simulink model "ofdm_txrx_supermimo_coded"  (Simulink 7.7)
settings: Solver=VariableStepDiscrete  SolverMode=SingleTasking  StartTime=0.0  StopTime=2*simOnly_simLength  RelTol=1e-3
PostLoadFcn:
  | ofdm_rx_supermimo_init
InitFcn:
  | ofdm_rx_supermimo_init
StartFcn:
  | ofdm_rx_supermimo_init
values from neighbouring files (symbols this model uses):
  ActionID_AFTransmit = 31    [ofdm_tx_supermimo_init.m]
  ActionID_Disabled = 0    [ofdm_tx_supermimo_init.m]
  ActionID_SetFlagA = 62    [ofdm_tx_supermimo_init.m]
  ActionID_SetFlagB = 61    [ofdm_tx_supermimo_init.m]
  BER_RAM_init_size = 2048    [ofdm_tx_supermimo_init.m]
  channel_h = [1 0; 1 0] * 0.3    [ofdm_tx_supermimo_init.m]
  CPLength = 16    [ofdm_tx_supermimo_init.m]
  longCorr_coef_i = [-4*real(LTS) 0]    [ofdm_rx_supermimo_init.m]
  longCorr_coef_q = [4*imag(LTS) 0]    [ofdm_rx_supermimo_init.m]
  max_CP_length = 16    [ofdm_tx_supermimo_init.m]
  max_num_baseRateSymbols = 31    [ofdm_tx_supermimo_init.m]
  max_num_subcarriers = 64    [ofdm_tx_supermimo_init.m]
  max_num_trainingSymbols = 15    [ofdm_tx_supermimo_init.m]
  max_numBytes = 16384    [ofdm_tx_supermimo_init.m]
  max_OFDM_symbols = 1023    [ofdm_tx_supermimo_init.m]
  modConstellation_qam16 = .75*[1 3 -1 -3]./3    [ofdm_tx_supermimo_init.m]
  modConstellation_qam64 = 0.875*[3 1 5 7 -3 -1 -5 -7]./7    [ofdm_tx_supermimo_init.m]
  modConstellation_qpsk = [1 -1]./sqrt(2)    [ofdm_tx_supermimo_init.m]
  numBaseRateSymbols = numBaseRateSymbols *2    [ofdm_tx_supermimo_init.m]
  numSubcarriers = 64    [ofdm_tx_supermimo_init.m]
  numTrainingSymbols = 2    [ofdm_tx_supermimo_init.m]
  pktTiming_count_payloadStart = pktTiming_count_lsStart + pktTiming_ls_num*pktTiming_ls_length    [ofdm_rx_supermimo_init.m]
  preamble = preamble_scale*[shortsyms_10 longsyms_2]    [ofdm_tx_supermimo_init.m]
  preamble_ROM = preamble_I_ROM + preamble_Q_ROM*2^16    [ofdm_tx_supermimo_init.m]
  RAM_init_size = max_numBytes    (= 16384)    [ofdm_tx_supermimo_init.m]
  RxDataScrambling_Seq = [40 198 78 63 82 173 102 245 48 111 172 115 147 230 216 93 72 65 62 2 205 242 122 90 128 83 105 97 73 10 5 252]    [ofdm_tx_supermimo_init.m]
  shortSymbol_time = shortSymbol_time(1:16).'    [ofdm_tx_supermimo_init.m]
  simOnly_numSamples = length(preamble)+( (numSubcarriers+CPLength)*(numTrainingSymbols + numBaseRateSymbols + numFullRateSymbols/2) )    [ofdm_tx_supermimo_init.m]
  train = [train train]    [ofdm_tx_supermimo_init.m]
  TxDataScrambling_Seq = [40 198 78 63 82 173 102 245 48 111 172 115 147 230 216 93 72 65 62 2 205 242 122 90 128 83 105 97 73 10 5 252]    [ofdm_tx_supermimo_init.m]
undefined symbols (not defined in the file or in its folder): AntA, AntB, BaseRate, Down, gcbh, numBuffs, numBytes, Up, xlBlockLoadCallback, xlGetNormalizedPeriod, xlipgetpartsetting
libraries: xbsIndex_r4 x5430
(part 9 of 49)

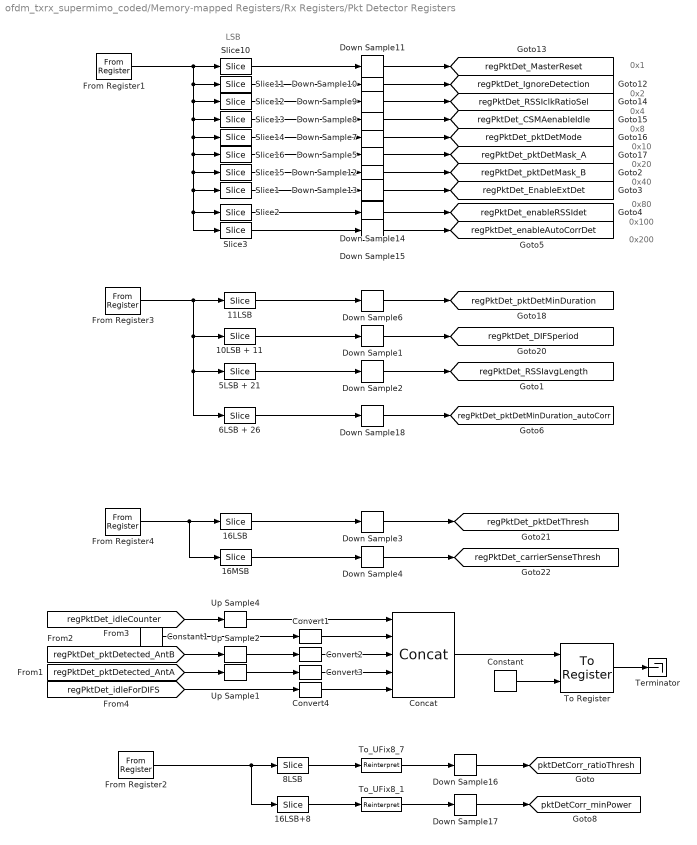
== system ofdm_txrx_supermimo_coded/Memory-mapped Registers/Rx Registers/Pkt Detector Registers ==
  note: 0x1
  note: 0x2
  note: 0x4
  note: 0x8
  note: 0x10
  note: LSB
  note: 0x20
  note: 0x40
  note: 0x80
  note: 0x100
  note: 0x200
  10LSB + 11 : xbsIndex_r4/Slice  nbits=10  boolean_output=off  mode=Lower Bit Location + Width  bit1=0  base1=MSB of Input  bit0=11  base0=LSB of Input  dbl_ovrd=off  block_type=slice
      settings: infoedit="Extracts a given range of bits from each input sample and presents it at the output.  The output type is ordinarily unsigned with binary point at zero, but can be Boolean when the slice is one bit wide.<P><P>Hardware notes: In hardware this block costs nothing."  block_version=VER_STRING_GOES_HERE
  11LSB : xbsIndex_r4/Slice  nbits=11  boolean_output=off  mode=Lower Bit Location + Width  bit1=0  base1=MSB of Input  bit0=0  base0=LSB of Input  dbl_ovrd=off  block_type=slice
      settings: infoedit="Extracts a given range of bits from each input sample and presents it at the output.  The output type is ordinarily unsigned with binary point at zero, but can be Boolean when the slice is one bit wide.<P><P>Hardware notes: In hardware this block costs nothing."  block_version=VER_STRING_GOES_HERE
  16LSB : xbsIndex_r4/Slice  nbits=16  boolean_output=off  mode=Lower Bit Location + Width  bit1=0  base1=MSB of Input  bit0=0  base0=LSB of Input  dbl_ovrd=off  block_type=slice
      settings: infoedit="Extracts a given range of bits from each input sample and presents it at the output.  The output type is ordinarily unsigned with binary point at zero, but can be Boolean when the slice is one bit wide.<P><P>Hardware notes: In hardware this block costs nothing."  block_version=VER_STRING_GOES_HERE
  16LSB+8 : xbsIndex_r4/Slice  nbits=16  boolean_output=off  mode=Lower Bit Location + Width  bit1=0  base1=MSB of Input  bit0=8  base0=LSB of Input  dbl_ovrd=off  block_type=slice
      settings: infoedit="Extracts a given range of bits from each input sample and presents it at the output.  The output type is ordinarily unsigned with binary point at zero, but can be Boolean when the slice is one bit wide.<P><P>Hardware notes: In hardware this block costs nothing."  block_version=VER_STRING_GOES_HERE
  16MSB : xbsIndex_r4/Slice  nbits=16  boolean_output=off  mode=Lower Bit Location + Width  bit1=0  base1=MSB of Input  bit0=16  base0=LSB of Input  dbl_ovrd=off  block_type=slice
      settings: infoedit="Extracts a given range of bits from each input sample and presents it at the output.  The output type is ordinarily unsigned with binary point at zero, but can be Boolean when the slice is one bit wide.<P><P>Hardware notes: In hardware this block costs nothing."  block_version=VER_STRING_GOES_HERE
  5LSB + 21 : xbsIndex_r4/Slice  nbits=5  boolean_output=off  mode=Lower Bit Location + Width  bit1=0  base1=MSB of Input  bit0=21  base0=LSB of Input  dbl_ovrd=off  block_type=slice
      settings: infoedit="Extracts a given range of bits from each input sample and presents it at the output.  The output type is ordinarily unsigned with binary point at zero, but can be Boolean when the slice is one bit wide.<P><P>Hardware notes: In hardware this block costs nothing."  block_version=VER_STRING_GOES_HERE
  6LSB + 26 : xbsIndex_r4/Slice  nbits=6  boolean_output=off  mode=Lower Bit Location + Width  bit1=0  base1=MSB of Input  bit0=26  base0=LSB of Input  dbl_ovrd=off  block_type=slice
      settings: block_version=VER_STRING_GOES_HERE
  8LSB : xbsIndex_r4/Slice  nbits=8  boolean_output=off  mode=Lower Bit Location + Width  bit1=0  base1=MSB of Input  bit0=0  base0=LSB of Input  dbl_ovrd=off  block_type=slice
      settings: infoedit="Extracts a given range of bits from each input sample and presents it at the output.  The output type is ordinarily unsigned with binary point at zero, but can be Boolean when the slice is one bit wide.<P><P>Hardware notes: In hardware this block costs nothing."  block_version=VER_STRING_GOES_HERE
  Concat : xbsIndex_r4/Concat  num_inputs=5  dbl_ovrd=off  block_type=concat
      settings: block_version=10.1.3
  Constant : xbsIndex_r4/Constant  const=1  gui_display_data_type=Fixed-point  arith_type=Boolean  n_bits=16  bin_pt=14  preci_type=Single  exp_width=8  frac_width=24  explicit_period=off  period=1  equ=P=C  opselect=C  inp2=PCIN>>17  opr=+  inp1=P  carry=CIN  dbl_ovrd=off  block_type=constant
  Constant1 : xbsIndex_r4/Constant  const=0  gui_display_data_type=Fixed-point  arith_type=Unsigned  n_bits=1  bin_pt=0  preci_type=Single  exp_width=8  frac_width=24  explicit_period=off  period=1  equ=P=C  opselect=C  inp2=PCIN>>17  opr=+  inp1=P  carry=CIN  dbl_ovrd=off  block_type=constant
  Convert{1..4} (4 alike) : xbsIndex_r4/Convert  gui_display_data_type=Fixed-point  arith_type=Unsigned  n_bits=1  bin_pt=0  float_type=Single  exp_bits=8  fraction_bits=24  quantization=Truncate  overflow=Wrap  en=off  latency=0  dbl_ovrd=off  pipeline=off  block_type=convert
      settings: block_version=10.1.3
  Down Sample{1..18} (18 alike) : xbsIndex_r4/Down Sample  sample_ratio=4  sample_phase="Last Value of Frame  (most efficient)"  en=off  latency=1  dbl_ovrd=off  block_type=dsamp
      settings: block_version=VER_STRING_GOES_HERE
  From Register1 : xbsIndex_r4/From Register  shared_memory_name='pktDet_controlBits'  init=pktDet_controlBits  period=1  ownership=Locally owned and initialized  gui_display_data_type=Fixed-point  arith_type=Unsigned  n_bits=32  bin_pt=0  preci_type=Single  dbl_ovrd=off  block_type=fromreg
      settings: AttributesFormatString=<< %<shared_memory_name> >>  infoedit="Register block that reads data to a shared memory register.  Delay of one sample period."
  From Register2 : xbsIndex_r4/From Register  shared_memory_name='pktDet_autoCorrParams'  init=rxReg_pktDetCorr  period=1  ownership=Locally owned and initialized  gui_display_data_type=Fixed-point  arith_type=Unsigned  n_bits=32  bin_pt=0  preci_type=Single  dbl_ovrd=off  block_type=fromreg
      settings: AttributesFormatString=<< %<shared_memory_name> >>  infoedit="Register block that reads data to a shared memory register.  Delay of one sample period."
  From Register3 : xbsIndex_r4/From Register  shared_memory_name='pktDet_durations'  init=(48 * 2^0) + (1000 * 2^11) + (16 * 2^21) + (1 * 2^26)  period=1  ownership=Locally owned and initialized  gui_display_data_type=Fixed-point  arith_type=Unsigned  n_bits=32  bin_pt=0  preci_type=Single  dbl_ovrd=off  block_type=fromreg
      settings: AttributesFormatString=<< %<shared_memory_name> >>  infoedit="Register block that reads data to a shared memory register.  Delay of one sample period."
  From Register4 : xbsIndex_r4/From Register  shared_memory_name='pktDet_thresholds'  init=(3000 * 2^0) + (3000 * 2^16)  period=1  ownership=Locally owned and initialized  gui_display_data_type=Fixed-point  arith_type=Unsigned  n_bits=32  bin_pt=0  preci_type=Single  dbl_ovrd=off  block_type=fromreg
      settings: AttributesFormatString=<< %<shared_memory_name> >>  infoedit="Register block that reads data to a shared memory register.  Delay of one sample period."
  Slice1 : xbsIndex_r4/Slice  nbits=1  boolean_output=on  mode=Lower Bit Location + Width  bit1=0  base1=MSB of Input  bit0=7  base0=LSB of Input  dbl_ovrd=off  block_type=slice
      settings: infoedit="Extracts a given range of bits from each input sample and presents it at the output.  The output type is ordinarily unsigned with binary point at zero, but can be Boolean when the slice is one bit wide.<P><P>Hardware notes: In hardware this block costs nothing."  block_version=VER_STRING_GOES_HERE
  Slice10 : xbsIndex_r4/Slice  nbits=1  boolean_output=on  mode=Lower Bit Location + Width  bit1=0  base1=MSB of Input  bit0=0  base0=LSB of Input  dbl_ovrd=off  block_type=slice
      settings: infoedit="Extracts a given range of bits from each input sample and presents it at the output.  The output type is ordinarily unsigned with binary point at zero, but can be Boolean when the slice is one bit wide.<P><P>Hardware notes: In hardware this block costs nothing."  block_version=VER_STRING_GOES_HERE
  Slice11 : xbsIndex_r4/Slice  nbits=1  boolean_output=on  mode=Lower Bit Location + Width  bit1=0  base1=MSB of Input  bit0=1  base0=LSB of Input  dbl_ovrd=off  block_type=slice
      settings: infoedit="Extracts a given range of bits from each input sample and presents it at the output.  The output type is ordinarily unsigned with binary point at zero, but can be Boolean when the slice is one bit wide.<P><P>Hardware notes: In hardware this block costs nothing."  block_version=VER_STRING_GOES_HERE
  Slice12 : xbsIndex_r4/Slice  nbits=1  boolean_output=on  mode=Lower Bit Location + Width  bit1=0  base1=MSB of Input  bit0=2  base0=LSB of Input  dbl_ovrd=off  block_type=slice
      settings: infoedit="Extracts a given range of bits from each input sample and presents it at the output.  The output type is ordinarily unsigned with binary point at zero, but can be Boolean when the slice is one bit wide.<P><P>Hardware notes: In hardware this block costs nothing."  block_version=VER_STRING_GOES_HERE
  Slice13 : xbsIndex_r4/Slice  nbits=1  boolean_output=on  mode=Lower Bit Location + Width  bit1=0  base1=MSB of Input  bit0=3  base0=LSB of Input  dbl_ovrd=off  block_type=slice
      settings: infoedit="Extracts a given range of bits from each input sample and presents it at the output.  The output type is ordinarily unsigned with binary point at zero, but can be Boolean when the slice is one bit wide.<P><P>Hardware notes: In hardware this block costs nothing."  block_version=VER_STRING_GOES_HERE
  Slice14 : xbsIndex_r4/Slice  nbits=1  boolean_output=on  mode=Lower Bit Location + Width  bit1=0  base1=MSB of Input  bit0=4  base0=LSB of Input  dbl_ovrd=off  block_type=slice
      settings: infoedit="Extracts a given range of bits from each input sample and presents it at the output.  The output type is ordinarily unsigned with binary point at zero, but can be Boolean when the slice is one bit wide.<P><P>Hardware notes: In hardware this block costs nothing."  block_version=VER_STRING_GOES_HERE
  Slice15 : xbsIndex_r4/Slice  nbits=1  boolean_output=on  mode=Lower Bit Location + Width  bit1=0  base1=MSB of Input  bit0=6  base0=LSB of Input  dbl_ovrd=off  block_type=slice
      settings: infoedit="Extracts a given range of bits from each input sample and presents it at the output.  The output type is ordinarily unsigned with binary point at zero, but can be Boolean when the slice is one bit wide.<P><P>Hardware notes: In hardware this block costs nothing."  block_version=VER_STRING_GOES_HERE
  Slice16 : xbsIndex_r4/Slice  nbits=1  boolean_output=on  mode=Lower Bit Location + Width  bit1=0  base1=MSB of Input  bit0=5  base0=LSB of Input  dbl_ovrd=off  block_type=slice
      settings: infoedit="Extracts a given range of bits from each input sample and presents it at the output.  The output type is ordinarily unsigned with binary point at zero, but can be Boolean when the slice is one bit wide.<P><P>Hardware notes: In hardware this block costs nothing."  block_version=VER_STRING_GOES_HERE
  Slice2 : xbsIndex_r4/Slice  nbits=1  boolean_output=on  mode=Lower Bit Location + Width  bit1=0  base1=MSB of Input  bit0=8  base0=LSB of Input  dbl_ovrd=off  block_type=slice
      settings: infoedit="Extracts a given range of bits from each input sample and presents it at the output.  The output type is ordinarily unsigned with binary point at zero, but can be Boolean when the slice is one bit wide.<P><P>Hardware notes: In hardware this block costs nothing."  block_version=VER_STRING_GOES_HERE
  Slice3 : xbsIndex_r4/Slice  nbits=1  boolean_output=on  mode=Lower Bit Location + Width  bit1=0  base1=MSB of Input  bit0=9  base0=LSB of Input  dbl_ovrd=off  block_type=slice
      settings: infoedit="Extracts a given range of bits from each input sample and presents it at the output.  The output type is ordinarily unsigned with binary point at zero, but can be Boolean when the slice is one bit wide.<P><P>Hardware notes: In hardware this block costs nothing."  block_version=VER_STRING_GOES_HERE
  Terminator : Terminator
  To Register : xbsIndex_r4/To Register  shared_memory_name='pktDet_status'  init=0  ownership=Locally owned and initialized  explicit_data_type=on  gui_display_data_type=Fixed-point  arith_type=Unsigned  n_bits=14  bin_pt=0  preci_type=Single  dbl_ovrd=off  block_type=toreg
      settings: AttributesFormatString=<< %<shared_memory_name> >>  infoedit="Register block that writes data to a shared memory register.  Delay of one sample period."  block_version=10.1.3
  To_UFix8_1 : xbsIndex_r4/Reinterpret  force_arith_type=on  arith_type=Unsigned  force_bin_pt=on  bin_pt=8  block_type=cast
      settings: infoedit="Changes type of samples without altering their binary representation.<P><P>Hardware notes: In hardware this block costs nothing.<P><P>Example:  Suppose the input is 6 bits wide, signed, with 2 fractional bits, and the output is forced to unsigned with 0 fractional bits.  Then an input of -2.0 (1110.00 in binary 2's complement) becomes an output of 56 (111000 in binary)."  block_version=VER_STRING_GOES_HERE
  To_UFix8_7 : xbsIndex_r4/Reinterpret  force_arith_type=on  arith_type=Unsigned  force_bin_pt=on  bin_pt=7  block_type=cast
      settings: infoedit="Changes type of samples without altering their binary representation.<P><P>Hardware notes: In hardware this block costs nothing.<P><P>Example:  Suppose the input is 6 bits wide, signed, with 2 fractional bits, and the output is forced to unsigned with 0 fractional bits.  Then an input of -2.0 (1110.00 in binary 2's complement) becomes an output of 56 (111000 in binary)."  block_version=VER_STRING_GOES_HERE
  Up Sample1 : xbsIndex_r4/Up Sample  sample_ratio=4  copy_samples=on  en=off  latency=0  dbl_ovrd=off  block_type=usamp
      settings: infoedit="Up samples input data.  Inserted values  can be zeros or copies of the most recent input sample.<P><P>Hardware notes: No hardware is needed if inserted values are copies of the input sample; otherwise, a mux and single bit flip-flop are used."  block_version=8.2
  Up Sample2 : xbsIndex_r4/Up Sample  sample_ratio=4  copy_samples=on  en=off  latency=0  dbl_ovrd=off  block_type=usamp
      settings: infoedit="Up samples input data.  Inserted values  can be zeros or copies of the most recent input sample.<P><P>Hardware notes: No hardware is needed if inserted values are copies of the input sample; otherwise, a mux and single bit flip-flop are used."  block_version=8.2
  Up Sample4 : xbsIndex_r4/Up Sample  sample_ratio=4  copy_samples=on  en=off  latency=0  dbl_ovrd=off  block_type=usamp
      settings: infoedit="Up samples input data.  Inserted values  can be zeros or copies of the most recent input sample.<P><P>Hardware notes: No hardware is needed if inserted values are copies of the input sample; otherwise, a mux and single bit flip-flop are used."  block_version=8.2
  tag scope: pktDetCorr_minPower=global, pktDetCorr_ratioThresh=global, regPktDet_CSMAenableIdle=global, regPktDet_DIFSperiod=global, regPktDet_EnableExtDet=global, regPktDet_IgnoreDetection=global, regPktDet_MasterReset=global, regPktDet_RSSIavgLength=global, regPktDet_RSSIclkRatioSel=global, regPktDet_carrierSenseThresh=global, regPktDet_enableAutoCorrDet=global, regPktDet_enableRSSIdet=global, regPktDet_pktDetMask_A=global, regPktDet_pktDetMask_B=global, regPktDet_pktDetMinDuration=global, regPktDet_pktDetMinDuration_autoCorr=global, regPktDet_pktDetMode=global, regPktDet_pktDetThresh=global
  wires:
  From Register1/1 -> Slice10/1, Slice11/1, Slice12/1, Slice13/1, Slice14/1, Slice16/1, Slice15/1, Slice1/1, Slice2/1, Slice3/1
  Slice10/1 -> Down Sample11/1
  Slice11/1 -> Down Sample10/1
  Slice12/1 -> Down Sample9/1
  Slice13/1 -> Down Sample8/1
  Slice14/1 -> Down Sample7/1
  From Register3/1 -> 11LSB/1, 10LSB + 11/1, 5LSB + 21/1, 6LSB + 26/1
  11LSB/1 -> Down Sample6/1
  10LSB + 11/1 -> Down Sample1/1
  Down Sample6/1 -> [regPktDet_pktDetMinDuration]
  Down Sample1/1 -> [regPktDet_DIFSperiod]
  5LSB + 21/1 -> Down Sample2/1
  Down Sample2/1 -> [regPktDet_RSSIavgLength]
  From Register4/1 -> 16LSB/1, 16MSB/1
  16LSB/1 -> Down Sample3/1
  Down Sample3/1 -> [regPktDet_pktDetThresh]
  16MSB/1 -> Down Sample4/1
  Down Sample4/1 -> [regPktDet_carrierSenseThresh]
  Down Sample5/1 -> [regPktDet_pktDetMask_A]
  Down Sample7/1 -> [regPktDet_pktDetMode]
  Down Sample8/1 -> [regPktDet_CSMAenableIdle]
  Down Sample9/1 -> [regPktDet_RSSIclkRatioSel]
  Down Sample10/1 -> [regPktDet_IgnoreDetection]
  Down Sample11/1 -> [regPktDet_MasterReset]
  Slice16/1 -> Down Sample5/1
  Slice15/1 -> Down Sample12/1
  Down Sample12/1 -> [regPktDet_pktDetMask_B]
  Constant/1 -> To Register/2
  Concat/1 -> To Register/1
  [regPktDet_idleForDIFS] -> Convert4/1
  [regPktDet_pktDetected_AntA] -> Up Sample1/1
  [regPktDet_pktDetected_AntB] -> Up Sample2/1
  Constant1/1 -> Convert1/1
  [regPktDet_idleCounter] -> Up Sample4/1
  Up Sample4/1 -> Concat/1
  Up Sample2/1 -> Convert2/1
  Up Sample1/1 -> Convert3/1
  Convert1/1 -> Concat/2
  Convert2/1 -> Concat/3
  Convert3/1 -> Concat/4
  Convert4/1 -> Concat/5
  To Register/1 -> Terminator/1
  Slice1/1 -> Down Sample13/1
  Down Sample13/1 -> [regPktDet_EnableExtDet]
  Slice2/1 -> Down Sample14/1
  Down Sample14/1 -> [regPktDet_enableRSSIdet]
  Slice3/1 -> Down Sample15/1
  Down Sample15/1 -> [regPktDet_enableAutoCorrDet]
  From Register2/1 -> 16LSB+8/1, 8LSB/1
  8LSB/1 -> To_UFix8_7/1
  To_UFix8_7/1 -> Down Sample16/1
  16LSB+8/1 -> To_UFix8_1/1
  Down Sample16/1 -> [pktDetCorr_ratioThresh]
  Down Sample17/1 -> [pktDetCorr_minPower]
  To_UFix8_1/1 -> Down Sample17/1
  6LSB + 26/1 -> Down Sample18/1
  Down Sample18/1 -> [regPktDet_pktDetMinDuration_autoCorr]

== system ofdm_txrx_supermimo_coded/Memory-mapped Registers/Rx Registers/UFix32  ==
  I : Inport
  Convert : xbsIndex_r4/Convert  gui_display_data_type=Fixed-point  arith_type=Unsigned  n_bits=32  bin_pt=0  float_type=Single  exp_bits=8  fraction_bits=24  quantization=Truncate  overflow=Wrap  en=off  latency=0  dbl_ovrd=off  pipeline=off  block_type=convert
  Reinterpret : xbsIndex_r4/Reinterpret  force_arith_type=on  arith_type=Unsigned  force_bin_pt=on  bin_pt=0  block_type=reinterpret
  O : Outport
  wires:
  Reinterpret/1 -> Convert/1
  Convert/1 -> O/1
  I/1 -> Reinterpret/1

== system ofdm_txrx_supermimo_coded/Memory-mapped Registers/Rx Registers/UFix32 1 ==
  I : Inport
  Convert : xbsIndex_r4/Convert  gui_display_data_type=Fixed-point  arith_type=Unsigned  n_bits=32  bin_pt=0  float_type=Single  exp_bits=8  fraction_bits=24  quantization=Truncate  overflow=Wrap  en=off  latency=0  dbl_ovrd=off  pipeline=off  block_type=convert
  Reinterpret : xbsIndex_r4/Reinterpret  force_arith_type=on  arith_type=Unsigned  force_bin_pt=on  bin_pt=0  block_type=reinterpret
  O : Outport
  wires:
  I/1 -> Reinterpret/1
  Convert/1 -> O/1
  Reinterpret/1 -> Convert/1

== system ofdm_txrx_supermimo_coded/Memory-mapped Registers/Rx Registers/UFix32 2 ==
  I : Inport
  Convert : xbsIndex_r4/Convert  gui_display_data_type=Fixed-point  arith_type=Unsigned  n_bits=32  bin_pt=0  float_type=Single  exp_bits=8  fraction_bits=24  quantization=Truncate  overflow=Wrap  en=off  latency=0  dbl_ovrd=off  pipeline=off  block_type=convert
  Reinterpret : xbsIndex_r4/Reinterpret  force_arith_type=on  arith_type=Unsigned  force_bin_pt=on  bin_pt=0  block_type=reinterpret
  O : Outport
  wires:
  Reinterpret/1 -> Convert/1
  Convert/1 -> O/1
  I/1 -> Reinterpret/1

== system ofdm_txrx_supermimo_coded/Memory-mapped Registers/Rx Registers/UFix32 3 ==
  I : Inport
  Convert : xbsIndex_r4/Convert  gui_display_data_type=Fixed-point  arith_type=Unsigned  n_bits=32  bin_pt=0  float_type=Single  exp_bits=8  fraction_bits=24  quantization=Truncate  overflow=Wrap  en=off  latency=0  dbl_ovrd=off  pipeline=off  block_type=convert
  Reinterpret : xbsIndex_r4/Reinterpret  force_arith_type=on  arith_type=Unsigned  force_bin_pt=on  bin_pt=0  block_type=reinterpret
  O : Outport
  wires:
  I/1 -> Reinterpret/1
  Convert/1 -> O/1
  Reinterpret/1 -> Convert/1

== system ofdm_txrx_supermimo_coded/Memory-mapped Registers/Rx Registers/UFix32 4 ==
  I : Inport
  Convert : xbsIndex_r4/Convert  gui_display_data_type=Fixed-point  arith_type=Unsigned  n_bits=32  bin_pt=0  float_type=Single  exp_bits=8  fraction_bits=24  quantization=Truncate  overflow=Wrap  en=off  latency=0  dbl_ovrd=off  pipeline=off  block_type=convert
  Reinterpret : xbsIndex_r4/Reinterpret  force_arith_type=on  arith_type=Unsigned  force_bin_pt=on  bin_pt=0  block_type=reinterpret
  O : Outport
  wires:
  Reinterpret/1 -> Convert/1
  Convert/1 -> O/1
  I/1 -> Reinterpret/1

== system ofdm_txrx_supermimo_coded/Memory-mapped Registers/Rx Registers/UFix32 5 ==
  I : Inport
  Convert : xbsIndex_r4/Convert  gui_display_data_type=Fixed-point  arith_type=Unsigned  n_bits=32  bin_pt=0  float_type=Single  exp_bits=8  fraction_bits=24  quantization=Truncate  overflow=Wrap  en=off  latency=0  dbl_ovrd=off  pipeline=off  block_type=convert
  Reinterpret : xbsIndex_r4/Reinterpret  force_arith_type=on  arith_type=Unsigned  force_bin_pt=on  bin_pt=0  block_type=reinterpret
  O : Outport
  wires:
  I/1 -> Reinterpret/1
  Convert/1 -> O/1
  Reinterpret/1 -> Convert/1

== system ofdm_txrx_supermimo_coded/Memory-mapped Registers/Rx Registers/UFix32 6 ==
  I : Inport
  Convert : xbsIndex_r4/Convert  gui_display_data_type=Fixed-point  arith_type=Unsigned  n_bits=32  bin_pt=0  float_type=Single  exp_bits=8  fraction_bits=24  quantization=Truncate  overflow=Wrap  en=off  latency=0  dbl_ovrd=off  pipeline=off  block_type=convert
  Reinterpret : xbsIndex_r4/Reinterpret  force_arith_type=on  arith_type=Unsigned  force_bin_pt=on  bin_pt=0  block_type=reinterpret
  O : Outport
  wires:
  Reinterpret/1 -> Convert/1
  Convert/1 -> O/1
  I/1 -> Reinterpret/1

== system ofdm_txrx_supermimo_coded/Memory-mapped Registers/Tx Registers/Control Bits Slices ==
  note: LSB
  note: 0x1
  note: 0x2
  note: 0x4
  note: 0x8
  note: 0xF0
  note: 0x200
  note: 0x400
  note: 0x100
  note: 0x800
  note: 0x1000
  note: 0x2000
  note: 0x4000
  note: 0x8000
  note: 0x1_0000
  note: 0x2_0000
  note: 0x4_0000
  note: 0x8_0000
  note: 0x3F0_0000
  32b : Inport
  Down Sample1 : xbsIndex_r4/Down Sample  sample_ratio=2  sample_phase="Last Value of Frame  (most efficient)"  en=off  latency=1  dbl_ovrd=off  block_type=dsamp
      settings: block_version=VER_STRING_GOES_HERE
  Down Sample3 : xbsIndex_r4/Down Sample  sample_ratio=2  sample_phase="Last Value of Frame  (most efficient)"  en=off  latency=1  dbl_ovrd=off  block_type=dsamp
      settings: block_version=VER_STRING_GOES_HERE
  Reinterpret : xbsIndex_r4/Reinterpret  force_arith_type=on  arith_type="Signed  (2's comp)"  force_bin_pt=off  bin_pt=13  block_type=reinterpret
      settings: infoedit="Changes signal type without altering the binary representation.   You can changed the signal between signed and unsigned, and relocate the binary point.<P><P>Hardware notes: In hardware this block costs nothing.<P><P>Example:  Suppose the input is 6 bits wide, signed, with 2 fractional bits, and the output is forced to unsigned with 0 fractional bits.  Then an input of -2.0 (1110.00 in binary 2's complement) becomes an output of 56 (111000 in binary)."  block_version=10.1
  Slice1 : xbsIndex_r4/Slice  nbits=1  boolean_output=on  mode=Lower Bit Location + Width  bit1=0  base1=MSB of Input  bit0=0  base0=LSB of Input  dbl_ovrd=off  block_type=slice
      settings: infoedit="Extracts a given range of bits from each input sample and presents it at the output.  The output type is ordinarily unsigned with binary point at zero, but can be Boolean when the slice is one bit wide.<P><P>Hardware notes: In hardware this block costs nothing."  block_version=VER_STRING_GOES_HERE
  Slice10 : xbsIndex_r4/Slice  nbits=1  boolean_output=on  mode=Lower Bit Location + Width  bit1=0  base1=MSB of Input  bit0=15  base0=LSB of Input  dbl_ovrd=off  block_type=slice
      settings: infoedit="Extracts a given range of bits from each input sample and presents it at the output.  The output type is ordinarily unsigned with binary point at zero, but can be Boolean when the slice is one bit wide.<P><P>Hardware notes: In hardware this block costs nothing."  block_version=VER_STRING_GOES_HERE
  Slice11 : xbsIndex_r4/Slice  nbits=1  boolean_output=off  mode=Lower Bit Location + Width  bit1=0  base1=MSB of Input  bit0=12  base0=LSB of Input  dbl_ovrd=off  block_type=slice
      settings: infoedit="Extracts a given range of bits from each input sample and presents it at the output.  The output type is ordinarily unsigned with binary point at zero, but can be Boolean when the slice is one bit wide.<P><P>Hardware notes: In hardware this block costs nothing."  block_version=VER_STRING_GOES_HERE
  Slice12 : xbsIndex_r4/Slice  nbits=1  boolean_output=off  mode=Lower Bit Location + Width  bit1=0  base1=MSB of Input  bit0=13  base0=LSB of Input  dbl_ovrd=off  block_type=slice
      settings: infoedit="Extracts a given range of bits from each input sample and presents it at the output.  The output type is ordinarily unsigned with binary point at zero, but can be Boolean when the slice is one bit wide.<P><P>Hardware notes: In hardware this block costs nothing."  block_version=VER_STRING_GOES_HERE
  Slice13 : xbsIndex_r4/Slice  nbits=1  boolean_output=off  mode=Lower Bit Location + Width  bit1=0  base1=MSB of Input  bit0=14  base0=LSB of Input  dbl_ovrd=off  block_type=slice
      settings: infoedit="Extracts a given range of bits from each input sample and presents it at the output.  The output type is ordinarily unsigned with binary point at zero, but can be Boolean when the slice is one bit wide.<P><P>Hardware notes: In hardware this block costs nothing."  block_version=VER_STRING_GOES_HERE
  Slice14 : xbsIndex_r4/Slice  nbits=1  boolean_output=on  mode=Lower Bit Location + Width  bit1=0  base1=MSB of Input  bit0=16  base0=LSB of Input  dbl_ovrd=off  block_type=slice
      settings: infoedit="Extracts a given range of bits from each input sample and presents it at the output.  The output type is ordinarily unsigned with binary point at zero, but can be Boolean when the slice is one bit wide.<P><P>Hardware notes: In hardware this block costs nothing."  block_version=VER_STRING_GOES_HERE
  Slice15 : xbsIndex_r4/Slice  nbits=1  boolean_output=off  mode=Lower Bit Location + Width  bit1=0  base1=MSB of Input  bit0=17  base0=LSB of Input  dbl_ovrd=off  block_type=slice
      settings: infoedit="Extracts a given range of bits from each input sample and presents it at the output.  The output type is ordinarily unsigned with binary point at zero, but can be Boolean when the slice is one bit wide.<P><P>Hardware notes: In hardware this block costs nothing."  block_version=VER_STRING_GOES_HERE
  Slice16 : xbsIndex_r4/Slice  nbits=1  boolean_output=off  mode=Lower Bit Location + Width  bit1=0  base1=MSB of Input  bit0=18  base0=LSB of Input  dbl_ovrd=off  block_type=slice
      settings: infoedit="Extracts a given range of bits from each input sample and presents it at the output.  The output type is ordinarily unsigned with binary point at zero, but can be Boolean when the slice is one bit wide.<P><P>Hardware notes: In hardware this block costs nothing."  block_version=VER_STRING_GOES_HERE
  Slice17 : xbsIndex_r4/Slice  nbits=1  boolean_output=off  mode=Lower Bit Location + Width  bit1=0  base1=MSB of Input  bit0=19  base0=LSB of Input  dbl_ovrd=off  block_type=slice
      settings: infoedit="Extracts a given range of bits from each input sample and presents it at the output.  The output type is ordinarily unsigned with binary point at zero, but can be Boolean when the slice is one bit wide.<P><P>Hardware notes: In hardware this block costs nothing."  block_version=VER_STRING_GOES_HERE
  Slice18 : xbsIndex_r4/Slice  nbits=6  boolean_output=off  mode=Lower Bit Location + Width  bit1=0  base1=MSB of Input  bit0=20  base0=LSB of Input  dbl_ovrd=off  block_type=slice
      settings: infoedit="Extracts a given range of bits from each input sample and presents it at the output.  The output type is ordinarily unsigned with binary point at zero, but can be Boolean when the slice is one bit wide.<P><P>Hardware notes: In hardware this block costs nothing."  block_version=VER_STRING_GOES_HERE
  Slice2 : xbsIndex_r4/Slice  nbits=1  boolean_output=on  mode=Lower Bit Location + Width  bit1=0  base1=MSB of Input  bit0=1  base0=LSB of Input  dbl_ovrd=off  block_type=slice
      settings: infoedit="Extracts a given range of bits from each input sample and presents it at the output.  The output type is ordinarily unsigned with binary point at zero, but can be Boolean when the slice is one bit wide.<P><P>Hardware notes: In hardware this block costs nothing."  block_version=VER_STRING_GOES_HERE
  Slice3 : xbsIndex_r4/Slice  nbits=1  boolean_output=on  mode=Lower Bit Location + Width  bit1=0  base1=MSB of Input  bit0=2  base0=LSB of Input  dbl_ovrd=off  block_type=slice
      settings: infoedit="Extracts a given range of bits from each input sample and presents it at the output.  The output type is ordinarily unsigned with binary point at zero, but can be Boolean when the slice is one bit wide.<P><P>Hardware notes: In hardware this block costs nothing."  block_version=VER_STRING_GOES_HERE
  Slice4 : xbsIndex_r4/Slice  nbits=1  boolean_output=on  mode=Lower Bit Location + Width  bit1=0  base1=MSB of Input  bit0=3  base0=LSB of Input  dbl_ovrd=off  block_type=slice
      settings: infoedit="Extracts a given range of bits from each input sample and presents it at the output.  The output type is ordinarily unsigned with binary point at zero, but can be Boolean when the slice is one bit wide.<P><P>Hardware notes: In hardware this block costs nothing."  block_version=VER_STRING_GOES_HERE
  Slice5 : xbsIndex_r4/Slice  nbits=4  boolean_output=off  mode=Lower Bit Location + Width  bit1=0  base1=MSB of Input  bit0=4  base0=LSB of Input  dbl_ovrd=off  block_type=slice
      settings: infoedit="Extracts a given range of bits from each input sample and presents it at the output.  The output type is ordinarily unsigned with binary point at zero, but can be Boolean when the slice is one bit wide.<P><P>Hardware notes: In hardware this block costs nothing."  block_version=VER_STRING_GOES_HERE
  Slice6 : xbsIndex_r4/Slice  nbits=1  boolean_output=off  mode=Lower Bit Location + Width  bit1=0  base1=MSB of Input  bit0=8  base0=LSB of Input  dbl_ovrd=off  block_type=slice
      settings: infoedit="Extracts a given range of bits from each input sample and presents it at the output.  The output type is ordinarily unsigned with binary point at zero, but can be Boolean when the slice is one bit wide.<P><P>Hardware notes: In hardware this block costs nothing."  block_version=VER_STRING_GOES_HERE
  Slice7 : xbsIndex_r4/Slice  nbits=1  boolean_output=off  mode=Lower Bit Location + Width  bit1=0  base1=MSB of Input  bit0=9  base0=LSB of Input  dbl_ovrd=off  block_type=slice
      settings: infoedit="Extracts a given range of bits from each input sample and presents it at the output.  The output type is ordinarily unsigned with binary point at zero, but can be Boolean when the slice is one bit wide.<P><P>Hardware notes: In hardware this block costs nothing."  block_version=VER_STRING_GOES_HERE
  Slice8 : xbsIndex_r4/Slice  nbits=1  boolean_output=off  mode=Lower Bit Location + Width  bit1=0  base1=MSB of Input  bit0=10  base0=LSB of Input  dbl_ovrd=off  block_type=slice
      settings: infoedit="Extracts a given range of bits from each input sample and presents it at the output.  The output type is ordinarily unsigned with binary point at zero, but can be Boolean when the slice is one bit wide.<P><P>Hardware notes: In hardware this block costs nothing."  block_version=VER_STRING_GOES_HERE
  Slice9 : xbsIndex_r4/Slice  nbits=1  boolean_output=on  mode=Lower Bit Location + Width  bit1=0  base1=MSB of Input  bit0=11  base0=LSB of Input  dbl_ovrd=off  block_type=slice
      settings: infoedit="Extracts a given range of bits from each input sample and presents it at the output.  The output type is ordinarily unsigned with binary point at zero, but can be Boolean when the slice is one bit wide.<P><P>Hardware notes: In hardware this block costs nothing."  block_version=VER_STRING_GOES_HERE
  tag scope: TxReg_Alamouti_Mode=global, TxReg_AntBPreambleShift=global, TxReg_DisableAntBPreamble=global, TxReg_Enable_ExtTxEn=global, TxReg_FilterSel=global, TxReg_PilotScrambling=global, TxReg_PostIFFTCycShift=global, TxReg_SISO_Mode=global, TxReg_SoftTxStart_TxEn=global, TxReg_SwapAntennas=global, TxReg_TxRunningOut_en0=global, TxReg_TxRunningOut_en1=global, regTx_AlwaysUsePreSpin=global, regTx_AutoTwoTx_En=global, regTx_CaptureRandomPayload=global, regTx_RandomPayload=global
  wires:
  Slice1/1 -> [TxReg_SISO_Mode]
  32b/1 -> Slice2/1, Slice5/1, Slice6/1, Slice7/1, Slice8/1, Slice9/1, Slice12/1, Slice13/1, Slice10/1, Slice14/1, Slice15/1, Slice16/1, Slice17/1, Slice18/1, Slice11/1, Slice4/1, Slice3/1, Slice1/1
  Slice3/1 -> Down Sample3/1
  Slice4/1 -> [TxReg_PilotScrambling]
  Slice5/1 -> Reinterpret/1
  Slice6/1 -> [regTx_RandomPayload]
  Slice2/1 -> [TxReg_Alamouti_Mode]
  Slice7/1 -> [TxReg_SwapAntennas]
  Slice8/1 -> [TxReg_SoftTxStart_TxEn]
  Slice9/1 -> [TxReg_Enable_ExtTxEn]
  Slice11/1 -> [regTx_AlwaysUsePreSpin]
  Slice12/1 -> [regTx_CaptureRandomPayload]
  Slice13/1 -> [regTx_AutoTwoTx_En]
  Slice10/1 -> [TxReg_TxRunningOut_en0]
  Slice14/1 -> [TxReg_TxRunningOut_en1]
  Slice15/1 -> [TxReg_FilterSel]
  Reinterpret/1 -> Down Sample1/1
  Down Sample1/1 -> [TxReg_AntBPreambleShift]
  Down Sample3/1 -> [TxReg_DisableAntBPreamble]
  Slice18/1 -> [TxReg_PostIFFTCycShift]

== system ofdm_txrx_supermimo_coded/Memory-mapped Registers/Tx Registers/UFix32 7 ==
  I : Inport
  Convert : xbsIndex_r4/Convert  gui_display_data_type=Fixed-point  arith_type=Unsigned  n_bits=32  bin_pt=0  float_type=Single  exp_bits=8  fraction_bits=24  quantization=Truncate  overflow=Wrap  en=off  latency=0  dbl_ovrd=off  pipeline=off  block_type=convert
  Reinterpret : xbsIndex_r4/Reinterpret  force_arith_type=on  arith_type=Unsigned  force_bin_pt=on  bin_pt=0  block_type=reinterpret
  O : Outport
  wires:
  Reinterpret/1 -> Convert/1
  Convert/1 -> O/1
  I/1 -> Reinterpret/1

== system ofdm_txrx_supermimo_coded/Memory-mapped Registers/TxRx Registers/InterruptControl ==
  Reg_8b : Inport
  LSB+0 : xbsIndex_r4/Slice  nbits=1  boolean_output=on  mode=Lower Bit Location + Width  bit1=0  base1=MSB of Input  bit0=0  base0=LSB of Input  dbl_ovrd=off  block_type=slice
      settings: infoedit="Extracts a given range of bits from each input sample and presents it at the output.  The output type is ordinarily unsigned with binary point at zero, but can be Boolean when the slice is one bit wide.<P><P>Hardware notes: In hardware this block costs nothing."  block_version=VER_STRING_GOES_HERE
  LSB+1 : xbsIndex_r4/Slice  nbits=1  boolean_output=on  mode=Lower Bit Location + Width  bit1=0  base1=MSB of Input  bit0=1  base0=LSB of Input  dbl_ovrd=off  block_type=slice
      settings: infoedit="Extracts a given range of bits from each input sample and presents it at the output.  The output type is ordinarily unsigned with binary point at zero, but can be Boolean when the slice is one bit wide.<P><P>Hardware notes: In hardware this block costs nothing."  block_version=VER_STRING_GOES_HERE
  LSB+2 : xbsIndex_r4/Slice  nbits=1  boolean_output=off  mode=Lower Bit Location + Width  bit1=0  base1=MSB of Input  bit0=2  base0=LSB of Input  dbl_ovrd=off  block_type=slice
      settings: infoedit="Extracts a given range of bits from each input sample and presents it at the output.  The output type is ordinarily unsigned with binary point at zero, but can be Boolean when the slice is one bit wide.<P><P>Hardware notes: In hardware this block costs nothing."  block_version=VER_STRING_GOES_HERE
  LSB+3 : xbsIndex_r4/Slice  nbits=1  boolean_output=off  mode=Lower Bit Location + Width  bit1=0  base1=MSB of Input  bit0=3  base0=LSB of Input  dbl_ovrd=off  block_type=slice
      settings: infoedit="Extracts a given range of bits from each input sample and presents it at the output.  The output type is ordinarily unsigned with binary point at zero, but can be Boolean when the slice is one bit wide.<P><P>Hardware notes: In hardware this block costs nothing."  block_version=VER_STRING_GOES_HERE
  LSB+4 : xbsIndex_r4/Slice  nbits=1  boolean_output=off  mode=Lower Bit Location + Width  bit1=0  base1=MSB of Input  bit0=4  base0=LSB of Input  dbl_ovrd=off  block_type=slice
      settings: infoedit="Extracts a given range of bits from each input sample and presents it at the output.  The output type is ordinarily unsigned with binary point at zero, but can be Boolean when the slice is one bit wide.<P><P>Hardware notes: In hardware this block costs nothing."  block_version=VER_STRING_GOES_HERE
  LSB+5 : xbsIndex_r4/Slice  nbits=1  boolean_output=off  mode=Lower Bit Location + Width  bit1=0  base1=MSB of Input  bit0=5  base0=LSB of Input  dbl_ovrd=off  block_type=slice
      settings: infoedit="Extracts a given range of bits from each input sample and presents it at the output.  The output type is ordinarily unsigned with binary point at zero, but can be Boolean when the slice is one bit wide.<P><P>Hardware notes: In hardware this block costs nothing."  block_version=VER_STRING_GOES_HERE
  LSB+6 : xbsIndex_r4/Slice  nbits=1  boolean_output=off  mode=Lower Bit Location + Width  bit1=0  base1=MSB of Input  bit0=6  base0=LSB of Input  dbl_ovrd=off  block_type=slice
      settings: infoedit="Extracts a given range of bits from each input sample and presents it at the output.  The output type is ordinarily unsigned with binary point at zero, but can be Boolean when the slice is one bit wide.<P><P>Hardware notes: In hardware this block costs nothing."  block_version=VER_STRING_GOES_HERE
  LSB+7 : xbsIndex_r4/Slice  nbits=1  boolean_output=off  mode=Lower Bit Location + Width  bit1=0  base1=MSB of Input  bit0=7  base0=LSB of Input  dbl_ovrd=off  block_type=slice
      settings: infoedit="Extracts a given range of bits from each input sample and presents it at the output.  The output type is ordinarily unsigned with binary point at zero, but can be Boolean when the slice is one bit wide.<P><P>Hardware notes: In hardware this block costs nothing."  block_version=VER_STRING_GOES_HERE
  Posedge : Subsystem
  Posedge1 : Subsystem
  Posedge2 : Subsystem
  tag scope: intrCtrl_RxBadHeader_en=global, intrCtrl_RxBadPkt_en=global, intrCtrl_RxGoodHeader_en=global, intrCtrl_RxGoodPkt_en=global, intrCtrl_RxHeaderInterrupts_rst=global, intrCtrl_RxPktInterrupts_rst=global, intrCtrl_TxDone_en=global, intrCtrl_TxDone_rst=global
  wires:
  LSB+0/1 -> Posedge/1(D)
  LSB+1/1 -> Posedge1/1(D)
  Reg_8b/1 -> LSB+0/1, LSB+1/1, LSB+2/1, LSB+4/1, LSB+5/1, LSB+6/1, LSB+7/1, LSB+3/1
  Posedge/1(Q) -> [intrCtrl_RxPktInterrupts_rst]
  Posedge1/1(Q) -> [intrCtrl_RxHeaderInterrupts_rst]
  LSB+4/1 -> [intrCtrl_RxBadPkt_en]
  LSB+5/1 -> [intrCtrl_RxGoodHeader_en]
  LSB+6/1 -> [intrCtrl_RxBadHeader_en]
  LSB+7/1 -> [intrCtrl_TxDone_en]
  LSB+3/1 -> [intrCtrl_RxGoodPkt_en]
  LSB+2/1 -> Posedge2/1(D)
  Posedge2/1(Q) -> [intrCtrl_TxDone_rst]

== system ofdm_txrx_supermimo_coded/OFDM Rx MIMO/ADC Inputs & Antenna Selection/Antenna Selection ==
  AntA_I : Inport
  AntA_Q : Inport  Port=2
  AntB_I : Inport  Port=3
  AntB_Q : Inport  Port=4
  PktDetReset : Inport  Port=5
  Ant Sel Logic : Subsystem
  AntA_Gains : Subsystem
  AntB_Gains : Subsystem
  Concat2 : xbsIndex_r4/Concat  num_inputs=3  dbl_ovrd=off  block_type=concat
      settings: block_version=VER_STRING_GOES_HERE
  Constant6 : xbsIndex_r4/Constant  const=0  gui_display_data_type=Fixed-point  arith_type=Unsigned  n_bits=1  bin_pt=0  preci_type=Single  exp_width=8  frac_width=24  explicit_period=off  period=1  equ=P=C  opselect=C  inp2=PCIN>>17  opr=+  inp1=P  carry=CIN  dbl_ovrd=off  block_type=constant
      settings: block_version=VER_STRING_GOES_HERE
  Mux : xbsIndex_r4/Mux  inputs=2  en=off  latency=1  precision=Full  arith_type=Unsigned  n_bits=16  bin_pt=14  quantization=Truncate  overflow=Wrap  dbl_ovrd=off  block_type=mux
      settings: block_version=8.2.02
  Mux1 : xbsIndex_r4/Mux  inputs=2  en=off  latency=1  precision=Full  arith_type=Unsigned  n_bits=16  bin_pt=14  quantization=Truncate  overflow=Wrap  dbl_ovrd=off  block_type=mux
      settings: block_version=8.2.02
  Posedge2 : Subsystem
  Register : xbsIndex_r4/Register  init=0  rst=off  en=off  dbl_ovrd=off  block_type=register
      settings: block_version=10.1
  Register1 : xbsIndex_r4/Register  init=0  rst=off  en=off  dbl_ovrd=off  block_type=register
      settings: block_version=10.1
  Register2 : xbsIndex_r4/Register  init=0  rst=off  en=off  dbl_ovrd=off  block_type=register
      settings: block_version=10.1
  Relational : xbsIndex_r4/Relational  mode=a>b  en=off  latency=0  dbl_ovrd=off  block_type=relational
      settings: block_version=8.2.02
  Rx_debug_antSel : xbsIndex_r4/Gateway Out  inherit_from_input=off  hdl_port=on  timing_constraint=None  locs_specified=off  LOCs={}  UseAsDAC=off  DACChannel='1'  block_type=gatewayout
      settings: infoedit="Gateway out block.  Converts Xilinx fixed point inputs into ouputs of type Simulink integer, double, or fixed point.<P><P>Hardware notes:  In hardware these blocks become top level output ports or are discarded, depending on how they are configured."  xl_area=[0 0 0 0 1 0 0]  block_version=VER_STRING_GOES_HERE
  Up Sample : xbsIndex_r4/Up Sample  sample_ratio=4  copy_samples=on  en=off  latency=0  dbl_ovrd=off  block_type=usamp
      settings: infoedit="Up samples input data.  Inserted values  can be zeros or copies of the most recent input sample.<P><P>Hardware notes: No hardware is needed if inserted values are copies of the input sample; otherwise, a mux and single bit flip-flop are used."  block_version=VER_STRING_GOES_HERE
  Up Sample1 : xbsIndex_r4/Up Sample  sample_ratio=4  copy_samples=on  en=off  latency=0  dbl_ovrd=off  block_type=usamp
      settings: infoedit="Up samples input data.  Inserted values  can be zeros or copies of the most recent input sample.<P><P>Hardware notes: No hardware is needed if inserted values are copies of the input sample; otherwise, a mux and single bit flip-flop are used."  block_version=VER_STRING_GOES_HERE
  Up Sample2 : xbsIndex_r4/Up Sample  sample_ratio=4  copy_samples=on  en=off  latency=0  dbl_ovrd=off  block_type=usamp
      settings: infoedit="Up samples input data.  Inserted values  can be zeros or copies of the most recent input sample.<P><P>Hardware notes: No hardware is needed if inserted values are copies of the input sample; otherwise, a mux and single bit flip-flop are used."  block_version=VER_STRING_GOES_HERE
  A I : Outport
  A Q : Outport  Port=2
  B I : Outport  Port=3
  B Q : Outport  Port=4
  A_swapped : Outport  Port=5
  tag scope: RxReg_RxGains=global, RxReg_RxGainsEn=global
  wires:
  AntB_I/1 -> Mux/3, Register2/1
  AntB_Q/1 -> Mux1/3, Register1/1
  AntA_I/1 -> Mux/2
  AntA_Q/1 -> Mux1/2
  Mux/1 -> A I/1
  Mux1/1 -> A Q/1
  PktDetReset/1 -> Up Sample1/1, AntB_Gains/1(Rst), AntA_Gains/1(Rst)
  AntA_Gains/2(GainVec) -> Relational/1, Concat2/3
  AntB_Gains/2(GainVec) -> Relational/2, Concat2/1
  Relational/1 -> Ant Sel Logic/1(G(B) < G(A))
  Ant Sel Logic/1(AntSel) -> Up Sample2/1, Mux/1, Mux1/1, A_swapped/1
  Up Sample2/1 -> Register/1
  Register2/1 -> B I/1
  Register1/1 -> B Q/1
  AntB_Gains/1(Vout) -> Ant Sel Logic/2(B_Vin)
  Concat2/1 -> Up Sample/1
  Up Sample/1 -> [RxReg_RxGains]
  Posedge2/1(Q) -> [RxReg_RxGainsEn]
  Constant6/1 -> Concat2/2
  Register/1 -> Rx_debug_antSel/1
  Up Sample1/1 -> Posedge2/1(D)

== system ofdm_txrx_supermimo_coded/OFDM Rx MIMO/AF Buffers/AF Buffers ==
  AntA_I : Inport
  AntA_Q : Inport  Port=2
  Payload : Inport  Port=3
  PktDone : Inport  Port=4
  Buffers : Subsystem
  Convert2 : xbsIndex_r4/Convert  gui_display_data_type=Fixed-point  arith_type=Boolean  n_bits=16  bin_pt=14  float_type=Single  exp_bits=8  fraction_bits=24  quantization=Truncate  overflow=Wrap  en=off  latency=0  dbl_ovrd=off  pipeline=off  block_type=convert
      settings: block_version=8.2.01
  Down Sample1 : xbsIndex_r4/Down Sample  sample_ratio=2  sample_phase="Last Value of Frame  (most efficient)"  en=off  latency=1  dbl_ovrd=off  block_type=dsamp
      settings: block_version=10.1.3
  Read Control : Subsystem
  Scaling : Subsystem
  Write Control : Subsystem
  I : Outport
  Q : Outport  Port=2
  AF Pream Index : Outport  Port=3
  AFTx Done : Outport  Port=4
  wires:
  Convert2/1 -> Write Control/2(AF Record En), Read Control/2(AF Record En)
  Down Sample1/1 -> Convert2/1
  [regRx_AF_SavePkt] -> Down Sample1/1
  Payload/1 -> Read Control/3(Payload)
  Write Control/2(Addr) -> Read Control/1(Wr Addr), Buffers/2(Wr_Addr)
  PktDone/1 -> Write Control/1(PktDone)
  Buffers/2(" Q") -> Scaling/2("Q ")
  Buffers/1(" I") -> Scaling/1("I ")
  AntA_Q/1 -> Buffers/6(Q)
  AntA_I/1 -> Buffers/5(I)
  Read Control/3(AF Pream Index) -> AF Pream Index/1
  Read Control/4(AFTx Done) -> AFTx Done/1
  Write Control/1(We) -> Buffers/1(Wr_En)
  Read Control/1(Rd Addr) -> Buffers/3(Rd_Addr)
  Read Control/2(New Read) -> Buffers/4(New Read)
  Scaling/1(I) -> I/1
  Scaling/2(Q) -> Q/1
